simulink model "TosAeb"  (R2021a)
settings: Solver=FixedStepDiscrete  StartTime=0.0  StopTime=inf  FixedStep=0.01  RelTol=1e-3
undefined symbols (not defined in the file or in its folder): ACCModSts, AccSts1, AEB_ObjectMovingOrientation, AEB_ObjectMovingState, CN_BTCActive, CN_CurrentGearR, CN_CurrentGearR_DHT, CN_PTCActive, CN_VDCActive, CN_VSC_EspOff, CN_VSC_FCW_AEB_Error, CN_VSC_FCW_AEB_NoDispay, CN_VSC_FCW_AEB_PerfmDgdtn, CN_VSC_FCW_AEB_TempUnAvlbl, CN_VSC_VehStandstill, CNaccelAvoidMax, CNaccelAvoidMin, CNbrakePedalPress, CNcomfortStrLeftAccelMax, CNcomfortStrLeftAccelMin, CNcomfortStrRightAccelMax, CNcomfortStrRightAccelMin, CNDrvDoorOpen, CNDrvModeEco, CNDrvModeNorm, CNDrvModeOther, CNDrvModeSport, CNDrvSBRreminder, CNNull, CNobjInvalidID, CNPassDoorOpen, CNPassSBRreminder, CNPEBSAccelNegInf, CNPEBSAccelNull, CNPEBSTimeInf, CNPEBSTimeNull, CNSTOPMAX, CNSTOPNULL, CNsubjWidth, CNSwtOFF, CNSwtON, CNTTCMAX, CNTTCNULL, CNVehConfigAEBCycNone, CNVehConfigAEBNone, CNVehConfigAEBPedNone, CNVehConfigFCWNone, CNVehConfigJANone, CollisionSeverity, FALSE, FCWSnvty, JACycObjCalcInfoDefaultParams, JAPedObjCalcInfoDefaultParams, JAVehObjCalcInfoDefaultParams, latCycObjCalcInfoDefaultParams, latPedObjCalcInfoDefaultParams, longObjCalcInfoDefaultParams, ModSts, movingStatus, ObjectType ... (+1112)
libraries: simulink x3031, AEBSFunctionLib x50, Ta_Library x33, AEBSStateMachineLib x23, Mylibrary x12, simulink_extras x7, ObjSelect_lib x3, dspstat3 x2
(part 27 of 102)

== system TosAeb/FcwAebJa_Step_sys/ALG_AEBSystem/ALG_AEBController/AEB_FuncImpManagementApp/FuncImpArbCalc/BrkFuncImpArbCalc/BrkFuncImpSuppCondCalc ==
  AEBpartModStsArb : Inport
  AEBfullModStsArb : Inport  Port=2
  EBAModStsArb : Inport  Port=3
  cycAEBpartModStsArb : Inport  Port=4
  cycAEBfullModStsArb : Inport  Port=5
  pedAEBfullModStsArb : Inport  Port=6
  JA_vehAEBModStsArb : Inport  Port=7
  JA_PTW_AEBModStsArb : Inport  Port=8
  JA_cycAEBModStsArb : Inport  Port=9
  JA_pedAEBModStsArb : Inport  Port=10
  AEBfullFuncImpSuppCondCalc : Subsystem
  AEBpartFuncImpSuppCondCalc : Subsystem
  Bus Creator : BusCreator  Inputs=10
  Bus Selector : BusSelector  OutputSignals=AEBpartModStsArb,AEBfullModStsArb,EBAModStsArb,cycAEBpartModStsArb,cycAEBfullModStsArb,pedAEBfullModStsArb,JA_vehAEBModStsArb,JA_PTW_AEBModStsArb,JA_cycAEBModStsArb,JA_pedAEBModStsArb
  Bus Selector1 : BusSelector  OutputSignals=AEBfullModStsArb,pedAEBfullModStsArb,cycAEBpartModStsArb,cycAEBfullModStsArb,JA_vehAEBModStsArb,EBAModStsArb,JA_PTW_AEBModStsArb,JA_cycAEBModStsArb,JA_pedAEBModStsArb
  Bus Selector2 : BusSelector  OutputSignals=AEBfullModStsArb,pedAEBfullModStsArb,cycAEBfullModStsArb,JA_vehAEBModStsArb,EBAModStsArb,JA_PTW_AEBModStsArb,JA_cycAEBModStsArb,JA_pedAEBModStsArb
  Bus Selector3 : BusSelector  OutputSignals=AEBfullModStsArb,pedAEBfullModStsArb,cycAEBfullModStsArb,EBAModStsArb,JA_PTW_AEBModStsArb,JA_cycAEBModStsArb,JA_pedAEBModStsArb
  Bus Selector4 : BusSelector  OutputSignals=pedAEBfullModStsArb,cycAEBfullModStsArb,EBAModStsArb,JA_PTW_AEBModStsArb,JA_cycAEBModStsArb,JA_pedAEBModStsArb
  Bus Selector5 : BusSelector  OutputSignals=pedAEBfullModStsArb,EBAModStsArb
  Bus Selector6 : BusSelector  OutputSignals=EBAModStsArb
  Bus Selector7 : BusSelector  OutputSignals=pedAEBfullModStsArb,cycAEBfullModStsArb,EBAModStsArb
  Bus Selector8 : BusSelector  OutputSignals=pedAEBfullModStsArb,cycAEBfullModStsArb,EBAModStsArb,JA_pedAEBModStsArb
  Bus Selector9 : BusSelector  OutputSignals=JA_cycAEBModStsArb,pedAEBfullModStsArb,JA_pedAEBModStsArb,cycAEBfullModStsArb,EBAModStsArb
  EBAFuncImpSuppCondCalc : Subsystem
  FCTBFuncImpSuppCondCalc : Subsystem
  JA_PTW_AEBfullFuncImpSuppCondCalc : Subsystem
  JA_cycAEBfullFuncImpSuppCondCalc : Subsystem
  JA_pedAEBfullFuncImpSuppCondCalc : Subsystem
  JA_vehAEBfullFuncImpSuppCondCalc : Subsystem
  cycAEBfullFuncImpSuppCondCalc : Subsystem
  cycAEBpartFuncImpSuppCondCalc : Subsystem
  pedAEBfullFuncImpSuppCondCalc : Subsystem
  FCTBFuncImpSuppCond : Outport
  AEBpartFuncImpSuppCond : Outport  Port=2
  cycAEBpartFuncImpSuppCond : Outport  Port=3
  JA_vehAEBfullFuncImpSuppCond : Outport  Port=4
  AEBfullFuncImpSuppCond : Outport  Port=5
  cycAEBfullFuncImpSuppCond : Outport  Port=6
  pedAEBfullFuncImpSuppCond : Outport  Port=7
  EBAFuncImpSuppCond : Outport  Port=8
  JA_pedAEBfullFuncImpSuppCond : Outport  Port=9
  JA_cycAEBfullFuncImpSuppCond : Outport  Port=10
  JA_PTW_AEBfullFuncImpSuppCond : Outport  Port=11
  wires:
  Bus Selector1/9 -> AEBpartFuncImpSuppCondCalc/9(JA_pedAEBfullModStsArb)  "<JA_pedAEBModStsArb>"
  Bus Selector1/8 -> AEBpartFuncImpSuppCondCalc/8(JA_cycAEBfullModStsArb)  "<JA_cycAEBModStsArb>"
  Bus Selector1/7 -> AEBpartFuncImpSuppCondCalc/7(JA_PTW_AEBfullModStsArb)  "<JA_PTW_AEBModStsArb>"
  Bus Selector2/8 -> cycAEBpartFuncImpSuppCondCalc/8(JA_pedAEBfullModStsArb)  "<JA_pedAEBModStsArb>"
  Bus Selector2/7 -> cycAEBpartFuncImpSuppCondCalc/7(JA_cycAEBfullModStsArb)  "<JA_cycAEBModStsArb>"
  Bus Selector2/6 -> cycAEBpartFuncImpSuppCondCalc/6(JA_PTW_AEBfullModStsArb)  "<JA_PTW_AEBModStsArb>"
  Bus Selector9/3 -> JA_PTW_AEBfullFuncImpSuppCondCalc/3(JA_pedAEBfullModStsArb)  "<JA_pedAEBModStsArb>"
  Bus Selector4/6 -> AEBfullFuncImpSuppCondCalc/6(JA_pedAEBfullModStsArb)  "<JA_pedAEBModStsArb>"
  Bus Selector4/5 -> AEBfullFuncImpSuppCondCalc/5(JA_cycAEBfullModStsArb)  "<JA_cycAEBModStsArb>"
  Bus Selector4/4 -> AEBfullFuncImpSuppCondCalc/4(JA_PTW_AEBfullModStsArb)  "<JA_PTW_AEBModStsArb>"
  AEBpartModStsArb/1 -> Bus Creator/1  "AEBpartModStsArb"
  Bus Selector/6 -> FCTBFuncImpSuppCondCalc/6(cycAEBpartModStsArb)  "<pedAEBfullModStsArb>"
  Bus Selector1/6 -> AEBpartFuncImpSuppCondCalc/6(EBAModStsArb)  "<EBAModStsArb>"
  Bus Selector/3 -> FCTBFuncImpSuppCondCalc/3(EBAModStsArb)  "<EBAModStsArb>"
  Bus Selector8/4 -> JA_cycAEBfullFuncImpSuppCondCalc/4(JA_pedAEBModStsArb)  "<JA_pedAEBModStsArb>"
  Bus Selector5/1 -> cycAEBfullFuncImpSuppCondCalc/1(pedAEBfullModStsArb)  "<pedAEBfullModStsArb>"
  Bus Selector/5 -> FCTBFuncImpSuppCondCalc/5(cycAEBfullModStsArb)  "<cycAEBfullModStsArb>"
  Bus Selector4/3 -> AEBfullFuncImpSuppCondCalc/3(EBAModStsArb)  "<EBAModStsArb>"
  pedAEBfullModStsArb/1 -> Bus Creator/6  "pedAEBfullModStsArb"
  Bus Selector2/4 -> cycAEBpartFuncImpSuppCondCalc/4(JA_vehAEBfullModStsArb)  "<JA_vehAEBModStsArb>"
  Bus Selector4/2 -> AEBfullFuncImpSuppCondCalc/2(cycAEBfullModStsArb)  "<cycAEBfullModStsArb>"
  Bus Selector9/4 -> JA_PTW_AEBfullFuncImpSuppCondCalc/4(cycAEBfullModStsArb)  "<cycAEBfullModStsArb>"
  Bus Selector9/2 -> JA_PTW_AEBfullFuncImpSuppCondCalc/2(pedAEBfullModStsArb)  "<pedAEBfullModStsArb>"
  Bus Selector3/4 -> JA_vehAEBfullFuncImpSuppCondCalc/4(EBAModStsArb)  "<EBAModStsArb>"
  Bus Selector5/2 -> cycAEBfullFuncImpSuppCondCalc/2(EBAModStsArb)  "<EBAModStsArb>"
  Bus Selector9/1 -> JA_PTW_AEBfullFuncImpSuppCondCalc/1(AEBfullModStsArb)  "<JA_cycAEBModStsArb>"
  JA_cycAEBfullFuncImpSuppCondCalc/1(JA_cycAEBfullFuncImpSuppCond) -> JA_cycAEBfullFuncImpSuppCond/1
  JA_PTW_AEBfullFuncImpSuppCondCalc/1(JA_PTW_AEBfullFuncImpSuppCond) -> JA_PTW_AEBfullFuncImpSuppCond/1
  Bus Selector7/1 -> JA_pedAEBfullFuncImpSuppCondCalc/1(cycAEBfullModStsArb)  "<pedAEBfullModStsArb>"
  Bus Selector1/5 -> AEBpartFuncImpSuppCondCalc/5(JA_vehAEBfullModStsArb)  "<JA_vehAEBModStsArb>"
  JA_pedAEBModStsArb/1 -> Bus Creator/10  "JA_pedAEBModStsArb"
  cycAEBfullModStsArb/1 -> Bus Creator/5  "cycAEBfullModStsArb"
  FCTBFuncImpSuppCondCalc/1(FCTBFuncImpSuppCond) -> FCTBFuncImpSuppCond/1
  Bus Selector7/3 -> JA_pedAEBfullFuncImpSuppCondCalc/3(EBAModStsArb)  "<EBAModStsArb>"
  Bus Selector/8 -> FCTBFuncImpSuppCondCalc/8(JA_PTW_AEBModStsArb)  "<JA_PTW_AEBModStsArb>"
  Bus Selector1/1 -> AEBpartFuncImpSuppCondCalc/1(AEBfullModStsArb)  "<AEBfullModStsArb>"
  Bus Selector1/2 -> AEBpartFuncImpSuppCondCalc/2(pedAEBfullModStsArb)  "<pedAEBfullModStsArb>"
  Bus Selector2/5 -> cycAEBpartFuncImpSuppCondCalc/5(EBAModStsArb)  "<EBAModStsArb>"
  JA_PTW_AEBModStsArb/1 -> Bus Creator/8  "JA_PTW_AEBModStsArb"
  Bus Selector1/4 -> AEBpartFuncImpSuppCondCalc/4(cycAEBfullModStsArb)  "<cycAEBfullModStsArb>"
  Bus Selector1/3 -> AEBpartFuncImpSuppCondCalc/3(cycAEBpartModStsArb)  "<cycAEBpartModStsArb>"
  Bus Selector7/2 -> JA_pedAEBfullFuncImpSuppCondCalc/2(pedAEBfullModStsArb)  "<cycAEBfullModStsArb>"
  Bus Selector/2 -> FCTBFuncImpSuppCondCalc/2(AEBfullModStsArb)  "<AEBfullModStsArb>"
  cycAEBfullFuncImpSuppCondCalc/1(cycAEBfullFuncImpSuppCond) -> cycAEBfullFuncImpSuppCond/1
  Bus Selector9/5 -> JA_PTW_AEBfullFuncImpSuppCondCalc/5(EBAModStsArb)  "<EBAModStsArb>"
  cycAEBpartModStsArb/1 -> Bus Creator/4  "cycAEBpartModStsArb"
  Bus Selector/10 -> FCTBFuncImpSuppCondCalc/10(JA_pedAEBModStsArb)  "<JA_pedAEBModStsArb>"
  Bus Selector4/1 -> AEBfullFuncImpSuppCondCalc/1(pedAEBfullModStsArb)  "<pedAEBfullModStsArb>"
  AEBfullModStsArb/1 -> Bus Creator/2  "AEBfullModStsArb"
  Bus Selector8/3 -> JA_cycAEBfullFuncImpSuppCondCalc/3(EBAModStsArb)  "<EBAModStsArb>"
  Bus Selector/4 -> FCTBFuncImpSuppCondCalc/4(pedAEBfullModStsArb)  "<cycAEBpartModStsArb>"
  Bus Selector/9 -> FCTBFuncImpSuppCondCalc/9(JA_cycAEBModStsArb)  "<JA_cycAEBModStsArb>"
  EBAModStsArb/1 -> Bus Creator/3  "EBAModStsArb"
  JA_cycAEBModStsArb/1 -> Bus Creator/9  "JA_cycAEBModStsArb"
  JA_pedAEBfullFuncImpSuppCondCalc/1(JA_pedAEBfullFuncImpSuppCond) -> JA_pedAEBfullFuncImpSuppCond/1
  Bus Selector2/2 -> cycAEBpartFuncImpSuppCondCalc/2(pedAEBfullModStsArb)  "<pedAEBfullModStsArb>"
  pedAEBfullFuncImpSuppCondCalc/1(pedAEBfullFuncImpSuppCond) -> pedAEBfullFuncImpSuppCond/1
  Bus Selector3/7 -> JA_vehAEBfullFuncImpSuppCondCalc/7(JA_pedAEBModStsArb)  "<JA_pedAEBModStsArb>"
  Bus Selector6/1 -> pedAEBfullFuncImpSuppCondCalc/1(EBAModStsArb)  "<EBAModStsArb>"
  JA_vehAEBModStsArb/1 -> Bus Creator/7  "JA_vehAEBModStsArb"
  Bus Selector3/2 -> JA_vehAEBfullFuncImpSuppCondCalc/2(cycAEBfullModStsArb)  "<pedAEBfullModStsArb>"
  Bus Selector3/1 -> JA_vehAEBfullFuncImpSuppCondCalc/1(AEBfullModStsArb)  "<AEBfullModStsArb>"
  Bus Selector3/3 -> JA_vehAEBfullFuncImpSuppCondCalc/3(pedAEBfullModStsArb)  "<cycAEBfullModStsArb>"
  Bus Selector3/5 -> JA_vehAEBfullFuncImpSuppCondCalc/5(JA_PTW_AEBModStsArb)  "<JA_PTW_AEBModStsArb>"
  cycAEBpartFuncImpSuppCondCalc/1(cycAEBpartFuncImpSuppCond) -> cycAEBpartFuncImpSuppCond/1
  AEBpartFuncImpSuppCondCalc/1(AEBpartFuncImpSuppCond) -> AEBpartFuncImpSuppCond/1
  JA_vehAEBfullFuncImpSuppCondCalc/1(JA_vehAEBfullFuncImpSuppCond) -> JA_vehAEBfullFuncImpSuppCond/1
  AEBfullFuncImpSuppCondCalc/1(AEBfullFuncImpSuppCond) -> AEBfullFuncImpSuppCond/1
  EBAFuncImpSuppCondCalc/1(EBAFuncImpSuppCond) -> EBAFuncImpSuppCond/1
  Bus Selector/7 -> FCTBFuncImpSuppCondCalc/7(JA_vehAEBfullModStsArb)  "<JA_vehAEBModStsArb>"
  Bus Selector3/6 -> JA_vehAEBfullFuncImpSuppCondCalc/6(JA_cycAEBModStsArb)  "<JA_cycAEBModStsArb>"
  Bus Selector2/1 -> cycAEBpartFuncImpSuppCondCalc/1(AEBfullModStsArb)  "<AEBfullModStsArb>"
  Bus Selector2/3 -> cycAEBpartFuncImpSuppCondCalc/3(cycAEBfullModStsArb)  "<cycAEBfullModStsArb>"
  Bus Creator/1 -> Bus Selector/1, Bus Selector1/1, Bus Selector2/1, Bus Selector3/1, Bus Selector4/1, Bus Selector9/1, Bus Selector8/1, Bus Selector7/1, Bus Selector5/1, Bus Selector6/1
  Bus Selector8/2 -> JA_cycAEBfullFuncImpSuppCondCalc/2(pedAEBfullModStsArb)  "<cycAEBfullModStsArb>"
  Bus Selector8/1 -> JA_cycAEBfullFuncImpSuppCondCalc/1(cycAEBfullModStsArb)  "<pedAEBfullModStsArb>"
  Bus Selector/1 -> FCTBFuncImpSuppCondCalc/1(AEBpartModStsArb)  "<AEBpartModStsArb>"

== system TosAeb/FcwAebJa_Step_sys/ALG_AEBSystem/ALG_AEBController/AEB_FuncImpManagementApp/FuncImpArbCalc/BrkFuncImpArbCalc/BrkFuncImpSuppCondCalc1 ==
  AEBModStsArb : Inport
  AEBfullFuncImpSuppCondCalc : Subsystem
  AEBpartFuncImpSuppCondCalc : Subsystem
  EBAFuncImpSuppCondCalc : Subsystem
  FCTBFuncImpSuppCondCalc : Subsystem
  JA_vehAEBfullFuncImpSuppCondCalc : Subsystem
  cycAEBfullFuncImpSuppCondCalc : Subsystem
  cycAEBpartFuncImpSuppCondCalc : Subsystem
  pedAEBfullFuncImpSuppCondCalc : Subsystem
  FCTBFuncImpSuppCond : Outport
  AEBpartFuncImpSuppCond : Outport  Port=2
  cycAEBpartFuncImpSuppCond : Outport  Port=3
  JA_vehAEBfullFuncImpSuppCond : Outport  Port=4
  AEBfullFuncImpSuppCond : Outport  Port=5
  cycAEBfullFuncImpSuppCond : Outport  Port=6
  pedAEBfullFuncImpSuppCond : Outport  Port=7
  EBAFuncImpSuppCond : Outport  Port=8
  wires:
  FCTBFuncImpSuppCondCalc/1(FCTBFuncImpSuppCond) -> FCTBFuncImpSuppCond/1  "FCTBFuncImpSupp"
  AEBpartFuncImpSuppCondCalc/1(AEBpartFuncImpSuppCond) -> AEBpartFuncImpSuppCond/1  "AEBpartFuncImpSupp"
  cycAEBpartFuncImpSuppCondCalc/1(cycAEBpartFuncImpSuppCond) -> cycAEBpartFuncImpSuppCond/1  "cycAEBpartFuncImpSupp"
  JA_vehAEBfullFuncImpSuppCondCalc/1(JA_vehAEBfullFuncImpSuppCond) -> JA_vehAEBfullFuncImpSuppCond/1  "JA_vehAEBfullFuncImpSupp"
  AEBfullFuncImpSuppCondCalc/1(AEBfullFuncImpSuppCond) -> AEBfullFuncImpSuppCond/1  "AEBfullFuncImpSupp"
  cycAEBfullFuncImpSuppCondCalc/1(cycAEBfullFuncImpSuppCond1) -> cycAEBfullFuncImpSuppCond/1  "cycAEBfullFuncImpSupp"
  pedAEBfullFuncImpSuppCondCalc/1(pedAEBfullFuncImpSuppCond) -> pedAEBfullFuncImpSuppCond/1  "pedAEBfullFuncImpSupp"
  EBAFuncImpSuppCondCalc/1(EBAFuncImpSuppCond) -> EBAFuncImpSuppCond/1  "EBAFuncImpSupp"

== system TosAeb/FcwAebJa_Step_sys/ALG_AEBSystem/ALG_AEBController/AEB_FuncImpManagementApp/FuncImpArbCalc/BrkFuncImpArbCalc1/BrkFumcActiveCondCalc ==
  AEBpartFuncImpArb : Inport
  cycAEBpartFuncImpArb : Inport  Port=2
  AEBfullFuncImpArb : Inport  Port=3
  JA_vehAEBfullFuncImpArb : Inport  Port=4
  cycAEBfullFuncImpArb : Inport  Port=5
  pedAEBfullFuncImpArb : Inport  Port=6
  EBAFuncImpArb : Inport  Port=7
  FCTBFuncImpArb : Inport  Port=8
  Compare To Constant{,1..7} (8 alike) : simulink/Logic and Bit Operations/Compare To Constant  relop===  const=ModSts.ActiveState  OutDataTypeStr=boolean
  Logical Operator : Logic  Operator=OR  Inputs=8  AllPortsSameDT=off  OutDataTypeStr=boolean
  brkFuncActvCond : Outport
  wires:
  AEBpartFuncImpArb/1 -> Compare To Constant/1
  cycAEBpartFuncImpArb/1 -> Compare To Constant1/1
  JA_vehAEBfullFuncImpArb/1 -> Compare To Constant3/1
  AEBfullFuncImpArb/1 -> Compare To Constant2/1
  EBAFuncImpArb/1 -> Compare To Constant6/1
  FCTBFuncImpArb/1 -> Compare To Constant7/1
  pedAEBfullFuncImpArb/1 -> Compare To Constant5/1
  cycAEBfullFuncImpArb/1 -> Compare To Constant4/1
  Compare To Constant3/1 -> Logical Operator/4
  Compare To Constant4/1 -> Logical Operator/5
  Compare To Constant2/1 -> Logical Operator/3
  Compare To Constant5/1 -> Logical Operator/6
  Compare To Constant1/1 -> Logical Operator/2
  Compare To Constant6/1 -> Logical Operator/7
  Compare To Constant/1 -> Logical Operator/1
  Compare To Constant7/1 -> Logical Operator/8
  Logical Operator/1 -> brkFuncActvCond/1

== system TosAeb/FcwAebJa_Step_sys/ALG_AEBSystem/ALG_AEBController/AEB_FuncImpManagementApp/FuncImpArbCalc/BrkFuncImpArbCalc1/BrkFuncImpAborCondCalc ==
  AEBModStsArb : Inport
  AEBfullFuncImpAborCondCalc : Subsystem
  AEBpartFuncImpAborCondCalc : Subsystem
  EBAFuncImpAborCondCalc : Subsystem
  FCTBFuncImpAborCondCalc : Subsystem
  JA_vehAEBfullFuncImpAborCondCalc : Subsystem
  cycAEBfullFuncImpAborCondCalc : Subsystem
  cycAEBpartFuncImpAborCondCalc : Subsystem
  pedAEBfullFuncImpAborCondCalc : Subsystem
  FCTBFuncImpAborCond : Outport
  AEBpartFuncImpAborCond : Outport  Port=2
  cycAEBpartFuncImpAborCond : Outport  Port=3
  JA_vehAEBfullFuncImpAborCond : Outport  Port=4
  AEBfullFuncImpAborCond : Outport  Port=5
  cycAEBfullFuncImpAborCond : Outport  Port=6
  pedAEBfullFuncImpAborCond : Outport  Port=7
  EBAFuncImpAborCond : Outport  Port=8
  wires:
  EBAFuncImpAborCondCalc/1(EBAFuncImpAborCond) -> EBAFuncImpAborCond/1  "EBAFuncImpAbor"
  pedAEBfullFuncImpAborCondCalc/1(pedAEBfullFuncImpAborCond) -> pedAEBfullFuncImpAborCond/1  "pedAEBfullFuncImpAbor"
  cycAEBfullFuncImpAborCondCalc/1(cycAEBfullFuncImpAborCond) -> cycAEBfullFuncImpAborCond/1  "cycAEBfullFuncImpAbor"
  AEBfullFuncImpAborCondCalc/1(AEBfullFuncImpAborCond) -> AEBfullFuncImpAborCond/1  "AEBfullFuncImpAbor"
  JA_vehAEBfullFuncImpAborCondCalc/1(JA_vehAEBfullFuncImpAborCond) -> JA_vehAEBfullFuncImpAborCond/1  "JA_vehAEBfullFuncImpAbor"
  cycAEBpartFuncImpAborCondCalc/1(cycAEBpartFuncImpAborCond) -> cycAEBpartFuncImpAborCond/1  "cycAEBpartFuncImpAbor"
  AEBpartFuncImpAborCondCalc/1(AEBpartFuncImpAborCond) -> AEBpartFuncImpAborCond/1  "AEBpartFuncImpAbor"
  FCTBFuncImpAborCondCalc/1(FCTBFuncImpAborCond) -> FCTBFuncImpAborCond/1  "FCTBFuncImpAbor"
  AEBModStsArb/1 -> FCTBFuncImpAborCondCalc/1(AEBModStsArb), AEBpartFuncImpAborCondCalc/1(AEBModStsArb), cycAEBpartFuncImpAborCondCalc/1(AEBModStsArb), JA_vehAEBfullFuncImpAborCondCalc/1(AEBModStsArb), AEBfullFuncImpAborCondCalc/1(AEBModStsArb), cycAEBfullFuncImpAborCondCalc/1(AEBModStsArb), pedAEBfullFuncImpAborCondCalc/1(AEBModStsArb)

== system TosAeb/FcwAebJa_Step_sys/ALG_AEBSystem/ALG_AEBController/AEB_FuncImpManagementApp/FuncImpArbCalc/BrkFuncImpArbCalc1/BrkFuncImpArbCalc ==
  AEBModStsArb : Inport
  brkFuncImpAborCondCond : Inport  Port=2
  FCTBModStsArb : Inport  Port=3
  AEBfullFuncImpArbCalc : Subsystem
  AEBpartFuncImpArbCalc : Subsystem
  Bus Creator : BusCreator  Inputs=2
  Bus Selector : BusSelector  OutputSignals=AEBModStsArb.vehAEBModStsArb.AEBpartModStsArb,BrkFuncImpAborCond.vehAEBpartFuncImpAborCond
  Bus Selector1 : BusSelector  OutputSignals=AEBModStsArb.latAEBModStsArb.cycAEBpartModStsArb,BrkFuncImpAborCond.cycAEBpartFuncImpAborCond
  Bus Selector2 : BusSelector  OutputSignals=AEBModStsArb.vehAEBModStsArb.AEBfullModStsArb,BrkFuncImpAborCond.vehAEBfullFuncImpAborCond
  Bus Selector3 : BusSelector  OutputSignals=AEBModStsArb.JA_vehAEBModStsArb.JA_vehAEBfullModStsArb,BrkFuncImpAborCond.JA_vehAEBfullFuncImpAborCond
  Bus Selector4 : BusSelector  OutputSignals=AEBModStsArb.latAEBModStsArb.cycAEBfullModStsArb,BrkFuncImpAborCond.cycAEBfullFuncImpAborCond
  Bus Selector5 : BusSelector  OutputSignals=AEBModStsArb.latAEBModStsArb.pedAEBfullModStsArb,BrkFuncImpAborCond.pedAEBfullFuncImpAborCond
  Bus Selector6 : BusSelector  OutputSignals=BrkFuncImpAborCond.FCTBFuncImpAborCond
  Bus Selector7 : BusSelector  OutputSignals=AEBModStsArb.vehAEBModStsArb.EBAModStsArb,BrkFuncImpAborCond.vehEBAFuncImpAborCond
  EBAFuncImpArbCalc : Subsystem
  FCTBFuncImpArbCalc : Subsystem
  JA_vehAEBfullFuncImpArbCalc : Subsystem
  cycAEBfullFuncImpArbCalc : Subsystem
  cycAEBpartFuncImpArbCalc : Subsystem
  pedAEBfullFuncImpArbCalc : Subsystem
  AEBpartFuncImpArb : Outport
  cycAEBpartFuncImpArb : Outport  Port=2
  AEBfullFuncImpArb : Outport  Port=3
  JA_vehAEBfullFuncImpArb : Outport  Port=4
  cycAEBfullFuncImpArb : Outport  Port=5
  pedAEBfullFuncImpArb : Outport  Port=6
  EBAFuncImpArb : Outport  Port=7
  FCTBFuncImpArb : Outport  Port=8
  wires:
  AEBModStsArb/1 -> Bus Creator/1  "AEBModStsArb"
  brkFuncImpAborCondCond/1 -> Bus Creator/2  "BrkFuncImpAborCond"
  Bus Selector/1 -> AEBpartFuncImpArbCalc/1(AEBpartModStsArb)  "<AEBpartModStsArb>"
  Bus Selector/2 -> AEBpartFuncImpArbCalc/2(AEBpartFuncImpAborCond)  "<vehAEBpartFuncImpAborCond>"
  AEBpartFuncImpArbCalc/1(AEBpartFuncImpArb) -> AEBpartFuncImpArb/1  "AEBpartFuncImpArb"
  Bus Selector1/2 -> cycAEBpartFuncImpArbCalc/2(cycAEBpartFuncImpAborCond)  "<cycAEBpartFuncImpAborCond>"
  Bus Selector1/1 -> cycAEBpartFuncImpArbCalc/1(cycAEBpartModStsArb)  "<cycAEBpartModStsArb>"
  Bus Selector2/2 -> AEBfullFuncImpArbCalc/2(AEBfullFuncImpAborCond)  "<vehAEBfullFuncImpAborCond>"
  Bus Selector3/2 -> JA_vehAEBfullFuncImpArbCalc/2(JA_vehAEBfullFuncImpAborCond)  "<JA_vehAEBfullFuncImpAborCond>"
  Bus Selector4/2 -> cycAEBfullFuncImpArbCalc/2(cycAEBfullFuncImpAborCond)  "<cycAEBfullFuncImpAborCond>"
  Bus Selector5/2 -> pedAEBfullFuncImpArbCalc/2(pedAEBfullFuncImpAborCond)  "<pedAEBfullFuncImpAborCond>"
  Bus Selector5/1 -> pedAEBfullFuncImpArbCalc/1(pedAEBfullModStsArb)  "<pedAEBfullModStsArb>"
  Bus Selector4/1 -> cycAEBfullFuncImpArbCalc/1(cycAEBfullModStsArb)  "<cycAEBfullModStsArb>"
  Bus Selector3/1 -> JA_vehAEBfullFuncImpArbCalc/1(JA_vehAEBfullfullModStsArb)  "<JA_vehAEBfullModStsArb>"
  Bus Selector2/1 -> AEBfullFuncImpArbCalc/1(AEBfullModStsArb)  "<AEBfullModStsArb>"
  Bus Selector7/2 -> EBAFuncImpArbCalc/2(EBAFuncImpAborCond)  "<vehEBAFuncImpAborCond>"
  Bus Selector7/1 -> EBAFuncImpArbCalc/1(EBAModStsArb)  "<EBAModStsArb>"
  Bus Creator/1 -> Bus Selector/1, Bus Selector1/1, Bus Selector2/1, Bus Selector3/1, Bus Selector4/1, Bus Selector5/1, Bus Selector6/1, Bus Selector7/1
  FCTBModStsArb/1 -> FCTBFuncImpArbCalc/1(FCTBModStsArb)
  Bus Selector6/1 -> FCTBFuncImpArbCalc/2(FCTBFuncImpAborCond)  "<FCTBFuncImpAborCond>"
  cycAEBpartFuncImpArbCalc/1(cycAEBpartFuncImpArb) -> cycAEBpartFuncImpArb/1  "cycAEBpartFuncImpArb"
  AEBfullFuncImpArbCalc/1(AEBfullFuncImpArb) -> AEBfullFuncImpArb/1  "AEBfullFuncImpArb"
  JA_vehAEBfullFuncImpArbCalc/1(JA_vehAEBfullFuncImpArb) -> JA_vehAEBfullFuncImpArb/1  "JA_AEBfullFuncImpArb"
  cycAEBfullFuncImpArbCalc/1(cycAEBfullFuncImpArb) -> cycAEBfullFuncImpArb/1  "cycAEBfullFuncImpArb"
  pedAEBfullFuncImpArbCalc/1(pedAEBfullFuncImpArb) -> pedAEBfullFuncImpArb/1  "pedAEBfullFuncImpArb"
  EBAFuncImpArbCalc/1(EBAFuncImpArb) -> EBAFuncImpArb/1  "EBAFuncImpArb"
  FCTBFuncImpArbCalc/1(FCTBFuncImpArb) -> FCTBFuncImpArb/1  "FCTBFuncImpArb"

== system TosAeb/FcwAebJa_Step_sys/ALG_AEBSystem/ALG_AEBController/AEB_FuncImpManagementApp/FuncImpArbCalc/BrkFuncImpArbCalc1/BrkFuncImpSuppCondCalc ==
  AEBModStsArb : Inport
  AEBfullFuncImpSuppCondCalc : Subsystem
  AEBpartFuncImpSuppCondCalc : Subsystem
  EBAFuncImpSuppCondCalc : Subsystem
  FCTBFuncImpSuppCondCalc : Subsystem
  JA_vehAEBfullFuncImpSuppCondCalc : Subsystem
  cycAEBfullFuncImpSuppCondCalc : Subsystem
  cycAEBpartFuncImpSuppCondCalc : Subsystem
  pedAEBfullFuncImpSuppCondCalc : Subsystem
  FCTBFuncImpSuppCond : Outport
  AEBpartFuncImpSuppCond : Outport  Port=2
  cycAEBpartFuncImpSuppCond : Outport  Port=3
  JA_vehAEBfullFuncImpSuppCond : Outport  Port=4
  AEBfullFuncImpSuppCond : Outport  Port=5
  cycAEBfullFuncImpSuppCond : Outport  Port=6
  pedAEBfullFuncImpSuppCond : Outport  Port=7
  EBAFuncImpSuppCond : Outport  Port=8
  wires:
  AEBModStsArb/1 -> pedAEBfullFuncImpSuppCondCalc/1(AEBModStsArb), cycAEBfullFuncImpSuppCondCalc/1(AEBModStsArb), AEBfullFuncImpSuppCondCalc/1(AEBModStsArb), JA_vehAEBfullFuncImpSuppCondCalc/1(AEBModStsArb), cycAEBpartFuncImpSuppCondCalc/1(AEBModStsArb), AEBpartFuncImpSuppCondCalc/1(AEBModStsArb), FCTBFuncImpSuppCondCalc/1(AEBModStsArb)
  FCTBFuncImpSuppCondCalc/1(FCTBFuncImpSuppCond) -> FCTBFuncImpSuppCond/1  "FCTBFuncImpSupp"
  AEBpartFuncImpSuppCondCalc/1(AEBpartFuncImpSuppCond) -> AEBpartFuncImpSuppCond/1  "AEBpartFuncImpSupp"
  cycAEBpartFuncImpSuppCondCalc/1(cycAEBpartFuncImpSuppCond) -> cycAEBpartFuncImpSuppCond/1  "cycAEBpartFuncImpSupp"
  JA_vehAEBfullFuncImpSuppCondCalc/1(JA_vehAEBfullFuncImpSuppCond) -> JA_vehAEBfullFuncImpSuppCond/1  "JA_vehAEBfullFuncImpSupp"
  AEBfullFuncImpSuppCondCalc/1(AEBfullFuncImpSuppCond) -> AEBfullFuncImpSuppCond/1  "AEBfullFuncImpSupp"
  cycAEBfullFuncImpSuppCondCalc/1(cycAEBfullFuncImpSuppCond) -> cycAEBfullFuncImpSuppCond/1  "cycAEBfullFuncImpSupp"
  pedAEBfullFuncImpSuppCondCalc/1(pedAEBfullFuncImpSuppCond) -> pedAEBfullFuncImpSuppCond/1  "pedAEBfullFuncImpSupp"
  EBAFuncImpSuppCondCalc/1(EBAFuncImpSuppCond) -> EBAFuncImpSuppCond/1  "EBAFuncImpSupp"

== system TosAeb/FcwAebJa_Step_sys/ALG_AEBSystem/ALG_AEBController/AEB_FuncImpManagementApp/FuncImpArbCalc/BrkReadyFuncImpArbCalc/BrkFuncImpSuppCondCalc ==
  prefillModStsArb : Inport
  ABAModStsArb : Inport  Port=2
  pedABAModStsArb : Inport  Port=3
  pedPrefillModStsArb : Inport  Port=4
  cycPrefillModStsArb : Inport  Port=5
  JA_vehPrefillModStsArb : Inport  Port=6
  JA_PTW_PrefillModStsArb : Inport  Port=7
  JA_cycPrefillModStsArb : Inport  Port=8
  JA_pedPrefillModStsArb : Inport  Port=9
  brkFuncActiveCond : Inport  Port=10
  Bus Creator : BusCreator  Inputs=10
  Bus Selector : BusSelector  OutputSignals=brkFuncActiveCond
  Bus Selector1 : BusSelector  OutputSignals=pedABAModStsArb
  Bus Selector10 : BusSelector  OutputSignals=ABAModStsArb,pedABAModStsArb
  Bus Selector2 : BusSelector  OutputSignals=brkFuncActiveCond,prefillModStsArb
  Bus Selector3 : BusSelector  OutputSignals=prefillModStsArb,pedPrefillModStsArb,brkFuncActiveCond
  Bus Selector5 : BusSelector  OutputSignals=prefillModStsArb,pedPrefillModStsArb,JA_PTW_PrefillModStsArb,JA_cycPrefillModStsArb,JA_pedPrefillModStsArb,brkFuncActiveCond
  Bus Selector6 : BusSelector  OutputSignals=prefillModStsArb,pedPrefillModStsArb,brkFuncActiveCond
  Bus Selector7 : BusSelector  OutputSignals=prefillModStsArb,pedPrefillModStsArb,JA_pedPrefillModStsArb,brkFuncActiveCond
  Bus Selector8 : BusSelector  OutputSignals=prefillModStsArb,pedPrefillModStsArb,JA_cycPrefillModStsArb,JA_pedPrefillModStsArb,brkFuncActiveCond
  Bus Selector9 : BusSelector  OutputSignals=prefillModStsArb,pedPrefillModStsArb,JA_cycPrefillModStsArb,JA_pedPrefillModStsArb,brkFuncActiveCond
  Compare To Constant{,2,4..5,11..15,17..21,23..24,26..28,30..32,34..35} (24 alike) : simulink/Logic and Bit Operations/Compare To Constant  relop===  const=ModSts.ActiveState  OutDataTypeStr=boolean
  Compare To Constant{1,3,6,16,22,25,29,33} (8 alike) : simulink/Logic and Bit Operations/Compare To Constant  relop===  const=TRUE  OutDataTypeStr=boolean
  Constant : Constant  Value=FALSE
  Logical Operator : Logic  Operator=OR  AllPortsSameDT=off  OutDataTypeStr=boolean
  Logical Operator1 : Logic  Operator=OR  Inputs=3  AllPortsSameDT=off  OutDataTypeStr=boolean
  Logical Operator3 : Logic  Operator=OR  Inputs=6  AllPortsSameDT=off  OutDataTypeStr=boolean
  Logical Operator4 : Logic  Operator=OR  Inputs=3  AllPortsSameDT=off  OutDataTypeStr=boolean
  Logical Operator5 : Logic  Operator=OR  Inputs=4  AllPortsSameDT=off  OutDataTypeStr=boolean
  Logical Operator6 : Logic  Operator=OR  Inputs=5  AllPortsSameDT=off  OutDataTypeStr=boolean
  Logical Operator7 : Logic  Operator=OR  Inputs=5  AllPortsSameDT=off  OutDataTypeStr=boolean
  Logical Operator8 : Logic  Operator=OR  AllPortsSameDT=off  OutDataTypeStr=boolean
  cycPrefillFuncImpSuppCond : Outport
  pedPrefillFuncImpSuppCond : Outport  Port=2
  prefillFuncImpSuppCond : Outport  Port=3
  ABAFuncImpSuppCond : Outport  Port=4
  pedABAFuncImpSuppCond : Outport  Port=5
  JA_vehPrefillFuncImpSuppCond : Outport  Port=6
  JA_pedPrefillFuncImpSuppCond : Outport  Port=7
  JA_cycPrefillFuncImpSuppCond : Outport  Port=8
  JA_PTW_PrefillFuncImpSuppCond : Outport  Port=9
  FCTB_PrefillFuncImpSuppCond : Outport  Port=10
  FCTB_ABAFuncImpSuppCond : Outport  Port=11
  wires:
  pedABAModStsArb/1 -> Bus Creator/3  "pedABAModStsArb"
  Compare To Constant19/1 -> Logical Operator5/1
  Bus Selector6/2 -> Compare To Constant18/1  "<pedPrefillModStsArb>"
  Bus Selector10/1 -> Compare To Constant34/1  "<ABAModStsArb>"
  Bus Selector7/1 -> Compare To Constant19/1  "<prefillModStsArb>"
  Bus Selector6/1 -> Compare To Constant17/1  "<prefillModStsArb>"
  Bus Selector9/2 -> Compare To Constant30/1  "<pedPrefillModStsArb>"
  Logical Operator3/1 -> JA_vehPrefillFuncImpSuppCond/1
  Bus Selector10/2 -> Compare To Constant35/1  "<pedABAModStsArb>"
  Compare To Constant27/1 -> Logical Operator6/3
  Compare To Constant24/1 -> Logical Operator5/3
  Logical Operator4/1 -> JA_pedPrefillFuncImpSuppCond/1
  Compare To Constant35/1 -> Logical Operator8/2
  Logical Operator7/1 -> FCTB_PrefillFuncImpSuppCond/1
  Logical Operator8/1 -> FCTB_ABAFuncImpSuppCond/1
  Compare To Constant34/1 -> Logical Operator8/1
  Bus Selector9/1 -> Compare To Constant26/1  "<prefillModStsArb>"
  Compare To Constant29/1 -> Logical Operator6/5
  Bus Selector8/4 -> Compare To Constant28/1  "<JA_pedPrefillModStsArb>"
  Compare To Constant16/1 -> Logical Operator3/6
  Bus Selector5/4 -> Compare To Constant14/1  "<JA_cycPrefillModStsArb>"
  Compare To Constant23/1 -> Logical Operator6/2
  Bus Selector7/3 -> Compare To Constant24/1  "<JA_pedPrefillModStsArb>"
  Bus Selector5/6 -> Compare To Constant16/1  "<brkFuncActiveCond>"
  Compare To Constant15/1 -> Logical Operator3/5
  Compare To Constant21/1 -> Logical Operator6/1
  Bus Selector5/3 -> Compare To Constant13/1  "<JA_PTW_PrefillModStsArb>"
  Compare To Constant17/1 -> Logical Operator4/1
  Compare To Constant18/1 -> Logical Operator4/2
  Compare To Constant26/1 -> Logical Operator7/1
  Bus Selector8/3 -> Compare To Constant27/1  "<JA_cycPrefillModStsArb>"
  Bus Selector5/5 -> Compare To Constant15/1  "<JA_pedPrefillModStsArb>"
  Logical Operator5/1 -> JA_cycPrefillFuncImpSuppCond/1
  Compare To Constant14/1 -> Logical Operator3/4
  Compare To Constant20/1 -> Logical Operator5/2
  Bus Selector6/3 -> Compare To Constant22/1  "<brkFuncActiveCond>"
  Compare To Constant28/1 -> Logical Operator6/4
  Compare To Constant33/1 -> Logical Operator7/5
  Bus Selector7/4 -> Compare To Constant25/1  "<brkFuncActiveCond>"
  Bus Selector7/2 -> Compare To Constant20/1  "<pedPrefillModStsArb>"
  Compare To Constant11/1 -> Logical Operator3/1
  Bus Creator/1 -> Bus Selector3/1, Bus Selector2/1, Bus Selector/1, Bus Selector1/1, Bus Selector5/1, Bus Selector6/1, Bus Selector7/1, Bus Selector8/1, Bus Selector9/1, Bus Selector10/1
  Compare To Constant22/1 -> Logical Operator4/3
  Compare To Constant25/1 -> Logical Operator5/4
  Compare To Constant13/1 -> Logical Operator3/3
  Bus Selector8/1 -> Compare To Constant21/1  "<prefillModStsArb>"
  Bus Selector8/2 -> Compare To Constant23/1  "<pedPrefillModStsArb>"
  Bus Selector8/5 -> Compare To Constant29/1  "<brkFuncActiveCond>"
  Logical Operator6/1 -> JA_PTW_PrefillFuncImpSuppCond/1
  Bus Selector5/2 -> Compare To Constant12/1  "<pedPrefillModStsArb>"
  Compare To Constant12/1 -> Logical Operator3/2
  Bus Selector5/1 -> Compare To Constant11/1  "<prefillModStsArb>"
  Compare To Constant32/1 -> Logical Operator7/4
  Bus Selector9/4 -> Compare To Constant32/1  "<JA_pedPrefillModStsArb>"
  Bus Selector9/5 -> Compare To Constant33/1  "<brkFuncActiveCond>"
  Bus Selector9/3 -> Compare To Constant31/1  "<JA_cycPrefillModStsArb>"
  Compare To Constant30/1 -> Logical Operator7/2
  Compare To Constant31/1 -> Logical Operator7/3
  cycPrefillModStsArb/1 -> Bus Creator/5  "cycPrefillModStsArb"
  JA_vehPrefillModStsArb/1 -> Bus Creator/6  "JA_vehPrefillModStsArb"
  ABAModStsArb/1 -> Bus Creator/2  "ABAModStsArb"
  pedPrefillModStsArb/1 -> Bus Creator/4  "pedPrefillModStsArb"
  prefillModStsArb/1 -> Bus Creator/1  "prefillModStsArb"
  brkFuncActiveCond/1 -> Bus Creator/10  "brkFuncActiveCond"
  JA_cycPrefillModStsArb/1 -> Bus Creator/8  "JA_cycPrefillModStsArb"
  JA_PTW_PrefillModStsArb/1 -> Bus Creator/7  "JA_PTW_PrefillModStsArb"
  JA_pedPrefillModStsArb/1 -> Bus Creator/9  "JA_pedPrefillModStsArb"
  Logical Operator1/1 -> cycPrefillFuncImpSuppCond/1
  Compare To Constant6/1 -> Logical Operator1/3
  Compare To Constant5/1 -> Logical Operator1/2
  Compare To Constant4/1 -> Logical Operator1/1
  Bus Selector3/3 -> Compare To Constant6/1  "<brkFuncActiveCond>"
  Bus Selector3/2 -> Compare To Constant5/1  "<pedPrefillModStsArb>"
  Bus Selector3/1 -> Compare To Constant4/1  "<prefillModStsArb>"
  Constant/1 -> pedABAFuncImpSuppCond/1
  Logical Operator/1 -> pedPrefillFuncImpSuppCond/1
  Compare To Constant2/1 -> Logical Operator/2
  Compare To Constant3/1 -> Logical Operator/1
  Bus Selector2/2 -> Compare To Constant2/1  "<prefillModStsArb>"
  Bus Selector2/1 -> Compare To Constant3/1  "<brkFuncActiveCond>"
  Compare To Constant/1 -> ABAFuncImpSuppCond/1
  Bus Selector1/1 -> Compare To Constant/1  "<pedABAModStsArb>"
  Compare To Constant1/1 -> prefillFuncImpSuppCond/1
  Bus Selector/1 -> Compare To Constant1/1  "<brkFuncActiveCond>"

== system TosAeb/FcwAebJa_Step_sys/ALG_AEBSystem/ALG_AEBController/AEB_FuncImpManagementApp/FuncImpArbCalc/BrkReadyFuncImpArbCalc/BrkReadyFuncActvCondCalc ==
  PrefillFuncImpArb : Inport
  ABAFuncImpArb : Inport  Port=2
  pedPrefillFuncImpArb : Inport  Port=3
  pedABAFuncImpArb : Inport  Port=4
  cycPrefillFuncImpArb : Inport  Port=5
  JA_vehPrefillFuncImpArb : Inport  Port=6
  JA_PTW_PrefillFuncImpArb : Inport  Port=7
  JA_cycPrefillFuncImpArb : Inport  Port=8
  JA_pedPrefillFuncImpArb : Inport  Port=9
  FCTB_PrefillFuncImpArb : Inport  Port=10
  FCTB_ABAFuncImpArb : Inport  Port=11
  Compare To Constant{,1..10} (11 alike) : simulink/Logic and Bit Operations/Compare To Constant  relop===  const=ModSts.ActiveState  OutDataTypeStr=boolean
  Logical Operator : Logic  Operator=OR  Inputs=11  AllPortsSameDT=off  OutDataTypeStr=boolean
  brkReadyActvCond : Outport
  wires:
  Compare To Constant10/1 -> Logical Operator/11
  Compare To Constant9/1 -> Logical Operator/10
  Compare To Constant8/1 -> Logical Operator/9
  Compare To Constant7/1 -> Logical Operator/8
  Compare To Constant6/1 -> Logical Operator/7
  JA_PTW_PrefillFuncImpArb/1 -> Compare To Constant6/1
  JA_cycPrefillFuncImpArb/1 -> Compare To Constant7/1
  JA_pedPrefillFuncImpArb/1 -> Compare To Constant8/1
  FCTB_PrefillFuncImpArb/1 -> Compare To Constant9/1
  FCTB_ABAFuncImpArb/1 -> Compare To Constant10/1
  Logical Operator/1 -> brkReadyActvCond/1
  Compare To Constant/1 -> Logical Operator/1
  Compare To Constant1/1 -> Logical Operator/2
  Compare To Constant2/1 -> Logical Operator/3
  Compare To Constant3/1 -> Logical Operator/4
  Compare To Constant5/1 -> Logical Operator/6
  Compare To Constant4/1 -> Logical Operator/5
  JA_vehPrefillFuncImpArb/1 -> Compare To Constant5/1
  cycPrefillFuncImpArb/1 -> Compare To Constant4/1
  pedABAFuncImpArb/1 -> Compare To Constant3/1
  pedPrefillFuncImpArb/1 -> Compare To Constant2/1
  ABAFuncImpArb/1 -> Compare To Constant1/1
  PrefillFuncImpArb/1 -> Compare To Constant/1

== system TosAeb/FcwAebJa_Step_sys/ALG_AEBSystem/ALG_AEBController/AEB_FuncImpManagementApp/FuncImpArbCalc/BrkReadyFuncImpArbCalc/BrkReadyFuncImpAborCondCalc ==
  prefillModStsArb : Inport
  ABAModStsArb : Inport  Port=2
  pedPrefillModStsArb : Inport  Port=3
  pedABAModStsArb : Inport  Port=4
  cycPrefillModStsArb : Inport  Port=5
  JA_vehPrefillModStsArb : Inport  Port=6
  JA_PTW_PrefillModStsArb : Inport  Port=7
  JA_cycPrefillModStsArb : Inport  Port=8
  JA_pedPrefillModStsArb : Inport  Port=9
  brkFuncActiveCond : Inport  Port=10
  Bus Creator : BusCreator  Inputs=10
  Bus Selector : BusSelector  OutputSignals=brkFuncActiveCond
  Bus Selector1 : BusSelector  OutputSignals=prefillModStsArb,brkFuncActiveCond
  Bus Selector10 : BusSelector  OutputSignals=ABAModStsArb,pedABAModStsArb
  Bus Selector2 : BusSelector  OutputSignals=prefillModStsArb,pedPrefillModStsArb,brkFuncActiveCond
  Bus Selector4 : BusSelector  OutputSignals=pedABAModStsArb
  Bus Selector5 : BusSelector  OutputSignals=prefillModStsArb,pedPrefillModStsArb,JA_PTW_PrefillModStsArb,JA_cycPrefillModStsArb,JA_pedPrefillModStsArb,brkFuncActiveCond
  Bus Selector6 : BusSelector  OutputSignals=prefillModStsArb,pedPrefillModStsArb,brkFuncActiveCond
  Bus Selector7 : BusSelector  OutputSignals=prefillModStsArb,pedPrefillModStsArb,JA_pedPrefillModStsArb,brkFuncActiveCond
  Bus Selector8 : BusSelector  OutputSignals=prefillModStsArb,pedPrefillModStsArb,JA_cycPrefillModStsArb,JA_pedPrefillModStsArb,brkFuncActiveCond
  Bus Selector9 : BusSelector  OutputSignals=prefillModStsArb,pedPrefillModStsArb,JA_cycPrefillModStsArb,JA_pedPrefillModStsArb,brkFuncActiveCond
  Compare To Constant{,3,6,16,22,25,29,33} (8 alike) : simulink/Logic and Bit Operations/Compare To Constant  relop===  const=TRUE  OutDataTypeStr=boolean
  Compare To Constant{1..2,4..5,11..15,17..21,23..24,26..28,30..32,34..35} (24 alike) : simulink/Logic and Bit Operations/Compare To Constant  relop===  const=ModSts.ActiveState  OutDataTypeStr=boolean
  Constant : Constant  Value=FALSE
  Logical Operator : Logic  Operator=OR  AllPortsSameDT=off  OutDataTypeStr=boolean
  Logical Operator1 : Logic  Operator=OR  Inputs=3  AllPortsSameDT=off  OutDataTypeStr=boolean
  Logical Operator3 : Logic  Operator=OR  Inputs=6  AllPortsSameDT=off  OutDataTypeStr=boolean
  Logical Operator4 : Logic  Operator=OR  Inputs=3  AllPortsSameDT=off  OutDataTypeStr=boolean
  Logical Operator5 : Logic  Operator=OR  Inputs=4  AllPortsSameDT=off  OutDataTypeStr=boolean
  Logical Operator6 : Logic  Operator=OR  Inputs=5  AllPortsSameDT=off  OutDataTypeStr=boolean
  Logical Operator7 : Logic  Operator=OR  Inputs=5  AllPortsSameDT=off  OutDataTypeStr=boolean
  Logical Operator8 : Logic  Operator=OR  AllPortsSameDT=off  OutDataTypeStr=boolean
  cycPrefillFuncImpAborCond : Outport
  pedPrefillFuncImpAborCond : Outport  Port=2
  prefillFuncImpAborCond : Outport  Port=3
  ABAFuncImpAborCond : Outport  Port=4
  pedABAFuncImpAborCond : Outport  Port=5
  JA_vehPrefillFuncImpAborCond : Outport  Port=6
  JA_pedPrefillFuncImpAborCond : Outport  Port=7
  JA_cycPrefillFuncImpAborCond : Outport  Port=8
  JA_PTW_PrefillFuncImpAborCond : Outport  Port=9
  FCTB_PrefillFuncImpAborCond : Outport  Port=10
  FCTB_ABAFuncImpAborCond : Outport  Port=11
  wires:
  Logical Operator8/1 -> FCTB_ABAFuncImpAborCond/1
  Compare To Constant34/1 -> Logical Operator8/1
  Compare To Constant35/1 -> Logical Operator8/2
  Bus Selector10/2 -> Compare To Constant35/1  "<pedABAModStsArb>"
  Bus Selector10/1 -> Compare To Constant34/1  "<ABAModStsArb>"
  Bus Creator/1 -> Bus Selector2/1, Bus Selector1/1, Bus Selector/1, Bus Selector5/1, Bus Selector6/1, Bus Selector7/1, Bus Selector8/1, Bus Selector9/1, Bus Selector10/1, Bus Selector4/1
  Compare To Constant31/1 -> Logical Operator7/3
  Bus Selector9/1 -> Compare To Constant26/1  "<prefillModStsArb>"
  Compare To Constant30/1 -> Logical Operator7/2
  Bus Selector9/2 -> Compare To Constant30/1  "<pedPrefillModStsArb>"
  Bus Selector9/3 -> Compare To Constant31/1  "<JA_cycPrefillModStsArb>"
  Compare To Constant26/1 -> Logical Operator7/1
  Compare To Constant33/1 -> Logical Operator7/5
  Bus Selector9/4 -> Compare To Constant32/1  "<JA_pedPrefillModStsArb>"
  Bus Selector9/5 -> Compare To Constant33/1  "<brkFuncActiveCond>"
  Compare To Constant32/1 -> Logical Operator7/4
  Logical Operator7/1 -> FCTB_PrefillFuncImpAborCond/1
  Logical Operator6/1 -> JA_PTW_PrefillFuncImpAborCond/1
  Compare To Constant28/1 -> Logical Operator6/4
  Bus Selector8/5 -> Compare To Constant29/1  "<brkFuncActiveCond>"
  Bus Selector8/4 -> Compare To Constant28/1  "<JA_pedPrefillModStsArb>"
  Compare To Constant29/1 -> Logical Operator6/5
  Compare To Constant21/1 -> Logical Operator6/1
  Bus Selector8/3 -> Compare To Constant27/1  "<JA_cycPrefillModStsArb>"
  Bus Selector8/2 -> Compare To Constant23/1  "<pedPrefillModStsArb>"
  Compare To Constant23/1 -> Logical Operator6/2
  Bus Selector8/1 -> Compare To Constant21/1  "<prefillModStsArb>"
  Compare To Constant27/1 -> Logical Operator6/3
  Bus Selector7/1 -> Compare To Constant19/1  "<prefillModStsArb>"
  Bus Selector7/2 -> Compare To Constant20/1  "<pedPrefillModStsArb>"
  Bus Selector7/3 -> Compare To Constant24/1  "<JA_pedPrefillModStsArb>"
  Bus Selector7/4 -> Compare To Constant25/1  "<brkFuncActiveCond>"
  Compare To Constant25/1 -> Logical Operator5/4
  Compare To Constant24/1 -> Logical Operator5/3
  Compare To Constant20/1 -> Logical Operator5/2
  Compare To Constant19/1 -> Logical Operator5/1
  Logical Operator5/1 -> JA_cycPrefillFuncImpAborCond/1
  Bus Selector6/1 -> Compare To Constant17/1  "<prefillModStsArb>"
  Bus Selector6/2 -> Compare To Constant18/1  "<pedPrefillModStsArb>"
  Bus Selector6/3 -> Compare To Constant22/1  "<brkFuncActiveCond>"
  Compare To Constant22/1 -> Logical Operator4/3
  Compare To Constant18/1 -> Logical Operator4/2
  Compare To Constant17/1 -> Logical Operator4/1
  Logical Operator4/1 -> JA_pedPrefillFuncImpAborCond/1
  Logical Operator3/1 -> JA_vehPrefillFuncImpAborCond/1
  Compare To Constant1/1 -> Logical Operator3/1
  Compare To Constant12/1 -> Logical Operator3/2
  Compare To Constant13/1 -> Logical Operator3/3
  Compare To Constant14/1 -> Logical Operator3/4
  Compare To Constant15/1 -> Logical Operator3/5
  Compare To Constant16/1 -> Logical Operator3/6
  Bus Selector5/6 -> Compare To Constant16/1  "<brkFuncActiveCond>"
  Bus Selector5/5 -> Compare To Constant15/1  "<JA_pedPrefillModStsArb>"
  Bus Selector5/4 -> Compare To Constant14/1  "<JA_cycPrefillModStsArb>"
  Bus Selector5/3 -> Compare To Constant13/1  "<JA_PTW_PrefillModStsArb>"
  Bus Selector5/2 -> Compare To Constant12/1  "<pedPrefillModStsArb>"
  Bus Selector5/1 -> Compare To Constant1/1  "<prefillModStsArb>"
  pedABAModStsArb/1 -> Bus Creator/4  "pedABAModStsArb"
  JA_pedPrefillModStsArb/1 -> Bus Creator/9  "JA_pedPrefillModStsArb"
  JA_cycPrefillModStsArb/1 -> Bus Creator/8  "JA_cycPrefillModStsArb"
  JA_PTW_PrefillModStsArb/1 -> Bus Creator/7  "JA_PTW_PrefillModStsArb"
  Compare To Constant11/1 -> ABAFuncImpAborCond/1
  Bus Selector4/1 -> Compare To Constant11/1  "<pedABAModStsArb>"
  Bus Selector2/1 -> Compare To Constant4/1  "<prefillModStsArb>"
  Bus Selector2/2 -> Compare To Constant5/1  "<pedPrefillModStsArb>"
  Bus Selector2/3 -> Compare To Constant6/1  "<brkFuncActiveCond>"
  Bus Selector1/2 -> Compare To Constant3/1  "<brkFuncActiveCond>"
  Bus Selector1/1 -> Compare To Constant2/1  "<prefillModStsArb>"
  Bus Selector/1 -> Compare To Constant/1  "<brkFuncActiveCond>"
  brkFuncActiveCond/1 -> Bus Creator/10  "brkFuncActiveCond"
  JA_vehPrefillModStsArb/1 -> Bus Creator/6  "JA_vehPrefillModStsArb"
  cycPrefillModStsArb/1 -> Bus Creator/5  "cycPrefillModStsArb"
  pedPrefillModStsArb/1 -> Bus Creator/3  "pedPrefillModStsArb"
  prefillModStsArb/1 -> Bus Creator/1  "prefillModStsArb"
  ABAModStsArb/1 -> Bus Creator/2  "ABAModStsArb"
  Logical Operator1/1 -> cycPrefillFuncImpAborCond/1
  Compare To Constant5/1 -> Logical Operator1/2
  Compare To Constant6/1 -> Logical Operator1/3
  Compare To Constant4/1 -> Logical Operator1/1
  Constant/1 -> pedABAFuncImpAborCond/1
  Logical Operator/1 -> pedPrefillFuncImpAborCond/1
  Compare To Constant3/1 -> Logical Operator/2
  Compare To Constant2/1 -> Logical Operator/1
  Compare To Constant/1 -> prefillFuncImpAborCond/1

== system TosAeb/FcwAebJa_Step_sys/ALG_AEBSystem/ALG_AEBController/AEB_FuncImpManagementApp/FuncImpArbCalc/BrkReadyFuncImpArbCalc/BrkReadyFuncImpArbCalc ==
  prefillModStsArb : Inport
  ABAModStsArb : Inport  Port=2
  pedPrefillModStsArb : Inport  Port=3
  pedABAModStsArb : Inport  Port=4
  cycPrefillModStsArb : Inport  Port=5
  JA_vehPrefillModStsArb : Inport  Port=6
  JA_PTW_PrefillModStsArb : Inport  Port=7
  JA_cycPrefillModStsArb : Inport  Port=8
  JA_pedPrefillModStsArb : Inport  Port=9
  FCTB_PrefillModStsArb : Inport  Port=10
  FCTB_ABAModStsArb : Inport  Port=11
  cycPrefillFuncImpAborCond : Inport  Port=12
  pedPrefillFuncImpAborCond : Inport  Port=13
  vehPrefillFuncImpAborCond : Inport  Port=14
  vehABAFuncImpAborCond : Inport  Port=15
  pedABAFuncImpAborCond : Inport  Port=16
  JA_vehPrefillFuncImpAborArb : Inport  Port=17
  JA_pedPrefillFuncImpAborArb : Inport  Port=18
  JA_cycPrefillFuncImpAborArb : Inport  Port=19
  JA_PTW_PrefillFuncImpAborArb : Inport  Port=20
  FCTB_PrefillFuncImpAborArb : Inport  Port=21
  FCTB_ABAFuncImpAborArb : Inport  Port=22
  ABAFuncImpArbCalc : Subsystem
  FCTB_ABAFuncImpArbCalc : Subsystem
  FCTB_PrefillFuncImpArbCalc : Subsystem
  JA_PTW_PrefillFuncImpArbCalc : Subsystem
  JA_cycPrefillFuncImpArbCalc : Subsystem
  JA_pedPrefillFuncImpArbCalc : Subsystem
  JA_vehPrefillFuncImpArbCalc : Subsystem
  PrefillFuncImpArbCalc : Subsystem
  cycPrefillFuncImpArbCalc : Subsystem
  pedABAFuncImpArbCalc : Subsystem
  pedPrefillFuncImpArbCalc : Subsystem
  prefillFuncImpArb : Outport
  ABAFuncImpArb : Outport  Port=2
  pedPrefillFuncImpArb : Outport  Port=3
  pedABAFuncImpArb : Outport  Port=4
  cycPrefillFuncImpArb : Outport  Port=5
  JA_vehPrefillFuncImpArb : Outport  Port=6
  JA_PTW_PrefillFuncImpArb : Outport  Port=7
  JA_cycPrefillFuncImpArb : Outport  Port=8
  JA_pedPrefillFuncImpArb : Outport  Port=9
  FCTB_PrefillFuncImpArb : Outport  Port=10
  FCTB_ABAFuncImpArb : Outport  Port=11
  wires:
  JA_pedPrefillFuncImpAborArb/1 -> JA_pedPrefillFuncImpArbCalc/2(JA_pedPrefillFuncImpAborCond)
  JA_PTW_PrefillFuncImpAborArb/1 -> JA_PTW_PrefillFuncImpArbCalc/2(JA_PTW_PrefillFuncImpAborCond)
  cycPrefillFuncImpAborCond/1 -> cycPrefillFuncImpArbCalc/2(cycPrefillFuncImpAborCond)
  pedABAFuncImpAborCond/1 -> pedABAFuncImpArbCalc/2(pedABAFuncImpAborCond)
  pedPrefillFuncImpAborCond/1 -> pedPrefillFuncImpArbCalc/2(pedPrefillFuncImpAborCond)
  vehABAFuncImpAborCond/1 -> ABAFuncImpArbCalc/2(ABAFuncImpAborCond)
  vehPrefillFuncImpAborCond/1 -> PrefillFuncImpArbCalc/2(prefillFuncImpAborCond)
  FCTB_ABAFuncImpArbCalc/1(FCTB_ABAFuncImpArb) -> FCTB_ABAFuncImpArb/1  "FCTB_ABAFuncImpArb"
  FCTB_PrefillFuncImpArbCalc/1(FCTB_PrefillFuncImpArb) -> FCTB_PrefillFuncImpArb/1  "FCTB_PrefillFuncImpArb"
  JA_pedPrefillFuncImpArbCalc/1(JA_pedPrefillFuncImpArb) -> JA_pedPrefillFuncImpArb/1  "JA_pedPrefillFuncImpArb"
  JA_cycPrefillFuncImpArbCalc/1(JA_cycPrefillFuncImpArb) -> JA_cycPrefillFuncImpArb/1  "JA_cycPrefillFuncImpArb"
  JA_PTW_PrefillFuncImpArbCalc/1(JA_PTW_PrefillFuncImpArb) -> JA_PTW_PrefillFuncImpArb/1  "JA_PTW_PrefillFuncImpArb"
  FCTB_ABAModStsArb/1 -> FCTB_ABAFuncImpArbCalc/1(FCTB_ABAModStsArb)
  FCTB_ABAFuncImpAborArb/1 -> FCTB_ABAFuncImpArbCalc/2(FCTB_ABAFuncImpAborCond)
  FCTB_PrefillFuncImpAborArb/1 -> FCTB_PrefillFuncImpArbCalc/2(FCTB_PrefillFuncImpAborCond)
  JA_cycPrefillFuncImpAborArb/1 -> JA_cycPrefillFuncImpArbCalc/2(JA_cycPrefillFuncImpAborCond)
  JA_vehPrefillFuncImpAborArb/1 -> JA_vehPrefillFuncImpArbCalc/2(JA_vehPrefillFuncImpAborCond)
  JA_pedPrefillModStsArb/1 -> JA_pedPrefillFuncImpArbCalc/1(JA_pedPrefillModStsArb)  "JA_pedPrefillModStsArb"
  FCTB_PrefillModStsArb/1 -> FCTB_PrefillFuncImpArbCalc/1(FCTB_PrefillModStsArb)
  JA_cycPrefillModStsArb/1 -> JA_cycPrefillFuncImpArbCalc/1(JA_cycPrefillModStsArb)  "JA_cycPrefillModStsArb"
  JA_PTW_PrefillModStsArb/1 -> JA_PTW_PrefillFuncImpArbCalc/1(JA_PTW_PrefillModStsArb)  "JA_PTW_PrefillModStsArb"
  pedABAModStsArb/1 -> pedABAFuncImpArbCalc/1(pedABAModStsArb)
  JA_vehPrefillModStsArb/1 -> JA_vehPrefillFuncImpArbCalc/1(JA_vehPrefillModStsArb)  "JA_vehPrefillModStsArb"
  cycPrefillModStsArb/1 -> cycPrefillFuncImpArbCalc/1(cycPrefillModStsArb)  "cycPrefillModStsArb"
  pedPrefillModStsArb/1 -> pedPrefillFuncImpArbCalc/1(pedPrefillModStsArb)  "pedPrefillModStsArb"
  ABAModStsArb/1 -> ABAFuncImpArbCalc/1(ABAModStsArb)  "ABAModStsArb"
  prefillModStsArb/1 -> PrefillFuncImpArbCalc/1(prefillModStsArb)  "prefillModStsArb"
  pedPrefillFuncImpArbCalc/1(pedPrefillFuncImpArb) -> pedPrefillFuncImpArb/1  "pedPrefillFuncImpArb"
  pedABAFuncImpArbCalc/1(pedABAFuncImpArb) -> pedABAFuncImpArb/1  "pedABAFuncImpArb"
  cycPrefillFuncImpArbCalc/1(cycPrefillFuncImpArb) -> cycPrefillFuncImpArb/1  "cycPrefillFuncImpArb"
  JA_vehPrefillFuncImpArbCalc/1(JA_vehPrefillFuncImpArb) -> JA_vehPrefillFuncImpArb/1  "JA_vehPrefillFuncImpArb"
  ABAFuncImpArbCalc/1(ABAFuncImpArb) -> ABAFuncImpArb/1  "ABAFuncImpArb"
  PrefillFuncImpArbCalc/1(prefillFuncImpArb) -> prefillFuncImpArb/1  "prefillFuncImpArb"
